simulink model "MX_Gateway"  (Simulink 9.0) (continued)
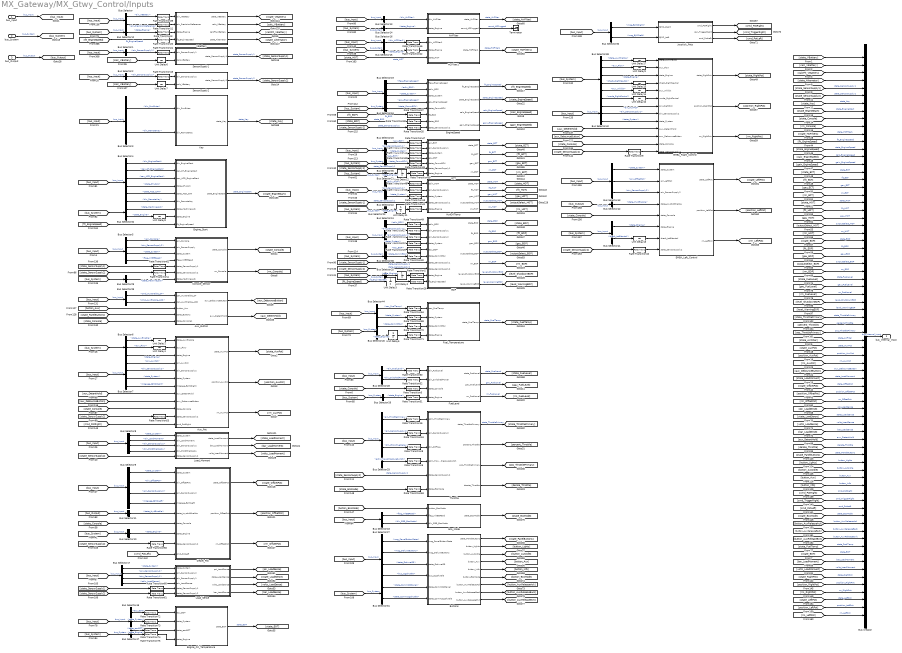
== system MX_Gateway/MX_Gtwy_Control/Inputs ==
  bus_Input : Inport
  bus_System : Inport  Port=2
  bus_Output : Inport  Port=3
  AirFilter : Subsystem  TreatAsAtomicUnit=on  SystemSampleTime=srate  RTWFileName=mod_Inputs
      settings: RTWSystemCode=Nonreusable function  RTWFileNameOpts=User specified
  Aux_Button : Subsystem
  Aux_Pot : Subsystem  TreatAsAtomicUnit=on  SystemSampleTime=srate  RTWFileName=mod_Inputs
      settings: RTWSystemCode=Nonreusable function  RTWFcnNameOpts=Use subsystem name  RTWFileNameOpts=User specified
  Bus Creator : BusCreator  Inputs=74
  Bus Selector : BusSelector  OutputSignals=bus_AnalogInputs.ain_VBattery,bus_AnalogInputs.ain_PrecisionReference
  Bus Selector1 : BusSelector  OutputSignals=bus_AnalogInputs.ain_SensorSupply1
  Bus Selector{10,12,14..15,21,23,25..26,47} (9 alike) : BusSelector  OutputSignals=state_Engine
  Bus Selector11 : BusSelector  OutputSignals=state_HydEnable
  Bus Selector13 : BusSelector  OutputSignals=bus_DigitalInputs.din_AirFilter
  Bus Selector16 : BusSelector  OutputSignals=bus_DigitalInputs.din_HCFilter1
  Bus Selector17 : BusSelector  OutputSignals=bus_Controller.state_System,bus_AnalogInputs.ain_SensorSupply2,bus_AnalogInputs.ain_SensorSupply1,bus_CombinedInputs.adin_LoadSense
  Bus Selector18 : BusSelector  OutputSignals=bus_Controller.flag_InitEERead,bus_CAN.bus_DeluxeG55.dlx_G55_EcoMode
  Bus Selector19 : BusSelector  OutputSignals=bus_FreqInputs.freq_EngineSpeed,bus_CombinedInputs.adin_EOP,bus_Controller.state_System,bus_CAN.bus_ECU.ecu_EngineSpeed
  Bus Selector2 : BusSelector  OutputSignals=bus_AnalogInputs.ain_SensorSupply2
  Bus Selector20 : BusSelector  OutputSignals=bus_AnalogInputs.ain_ECT,bus_AnalogInputs.ain_SensorSupply1,bus_Controller.state_System,bus_CAN.bus_ECU.ecu_ECT
  Bus Selector22 : BusSelector  OutputSignals=bus_CombinedInputs.adin_HOT,bus_AnalogInputs.ain_SensorSupply1,bus_Controller.state_System
  Bus Selector24 : BusSelector  OutputSignals=bus_CombinedInputs.adin_EOP,bus_AnalogInputs.ain_SensorSupply1,bus_AnalogInputs.ain_SensorSupply2,bus_Controller.state_System,bus_CAN.bus_ECU.ecu_EOP
  Bus Selector27 : BusSelector  OutputSignals=bus_CAN.bus_StdDisplay.msg_PanelButtonState,bus_CAN.bus_DeluxeG5.msg_DeluxeButtons,bus_CAN.bus_DeluxeG5.state_DeluxeG5,bus_CAN.bus_JogShuttle
  Bus Selector28 : BusSelector  OutputSignals=bus_AnalogInputs.ain_FuelLevel,bus_AnalogInputs.ain_SwitchedPower
  Bus Selector29 : BusSelector  OutputSignals=state_MachineLock
  Bus Selector3 : BusSelector  OutputSignals=bus_DigitalInputs.din_RunEnter,bus_DigitalInputs.din_RemoteKey
  Bus Selector30 : BusSelector  OutputSignals=bus_CAN.bus_CANJoystickRight.msg_BJM2Right,bus_CAN.bus_CANJoystickLeft.msg_BJM2Left
  Bus Selector31 : BusSelector  OutputSignals=state_CommStdPanel,state_CommJogShuttle
  Bus Selector32 : BusSelector  OutputSignals=state_MachineLock,state_Engine
  Bus Selector33 : BusSelector  OutputSignals=bus_AnalogInputs.ain_ThrottlePrimary,bus_AnalogInputs.ain_SensorSupply2,bus_DigitalInputs.din_GlowPlugRelay,bus_CombinedInputs.adin_TravelPressureSwitch
  Bus Selector34 : BusSelector  OutputSignals=state_CommECU
  Bus Selector35 : BusSelector  OutputSignals=state_CommECU
  Bus Selector36 : BusSelector  OutputSignals=state_CommECU
  Bus Selector38 : BusSelector  OutputSignals=state_AuxEnable,aux_Flow,state_Engine,check_RightNeutral,aux_Inhibit,mode_RightControl
  Bus Selector39 : BusSelector  OutputSignals=bus_CAN.bus_ServiceTool.cmd_AuxEnable_ST,bus_CAN.bus_ACD.cmd_AuxEnable_ACD
  Bus Selector4 : BusSelector  OutputSignals=bus_DigitalInputs.din_EngineStart,bus_CAN.bus_ServiceTool.can_ST_EngineStart,bus_CAN.bus_ACD.can_ACD_EngineStart,bus_CAN.bus_ServiceTool.state_ST_CAN,bus_CAN.bus_ACD.state_ACD_CAN,bus_DigitalInputs.din_RemoteKey
  Bus Selector40 : BusSelector  OutputSignals=bus_AnalogInputs.ain_AuxPot,bus_AnalogInputs.ain_SensorSupply2,bus_Controller.state_System
  Bus Selector41 : BusSelector  OutputSignals=state_Engine,check_LeftNeutral
  Bus Selector42 : BusSelector  OutputSignals=state_HydEnable
  Bus Selector43 : BusSelector  OutputSignals=bus_Controller.state_System,bus_AnalogInputs.ain_OffsetPot,bus_AnalogInputs.ain_SensorSupply2
  Bus Selector44 : BusSelector  OutputSignals=bus_CAN.bus_ECU.can_FuelTemp,bus_Controller.state_System
  Bus Selector45 : BusSelector  OutputSignals=state_CommECU,state_Engine
  Bus Selector46 : BusSelector  OutputSignals=bus_CAN.bus_ECU.ecu_EOT,bus_Controller.state_System,bus_CAN.bus_ECU.state_ecuECT
  Bus Selector5 : BusSelector  OutputSignals=bus_AnalogInputs.ain_Console,bus_AnalogInputs.ain_SensorSupply2,bus_Controller.state_System,bus_Controller.flag_InitEERead
  Bus Selector6 : BusSelector  OutputSignals=state_AuxEnable,aux_Flow,state_Engine
  Bus Selector7 : BusSelector  OutputSignals=bus_AnalogInputs.ain_AuxPot,bus_AnalogInputs.ain_SensorSupply2,bus_Controller.state_System,bus_CAN.bus_CANJoystickRight.msgAge_BJM2Right
  Bus Selector8 : BusSelector  OutputSignals=bus_Controller.state_System,bus_AnalogInputs.ain_LoadMoment,bus_AnalogInputs.ain_SensorSupply2,bus_DigitalInputs.din_OWDEnableSw
  Bus Selector9 : BusSelector  OutputSignals=bus_Controller.state_System,bus_AnalogInputs.ain_OffsetPot,bus_AnalogInputs.ain_SensorSupply2,bus_CAN.bus_CANJoystickLeft.msgAge_BJM2Left
  Buttons : Subsystem  TreatAsAtomicUnit=on  SystemSampleTime=srate  RTWFileName=mod_Inputs
      settings: RTWSystemCode=Nonreusable function  RTWFileNameOpts=User specified
  Console_Sensor : Subsystem  TreatAsAtomicUnit=on  SystemSampleTime=srate  RTWFileName=mod_Inputs
      settings: RTWSystemCode=Nonreusable function  RTWFcnNameOpts=Use subsystem name  RTWFileNameOpts=User specified
  ECT : Subsystem  TreatAsAtomicUnit=on  SystemSampleTime=srate100  RTWFileName=mod_Inputs
      settings: RTWSystemCode=Nonreusable function  RTWFcnNameOpts=Use subsystem name  RTWFileNameOpts=User specified
  EMEA_Left_Control : Subsystem  SystemSampleTime=srate  RTWFileName=mod_Inputs
      settings: RTWSystemCode=Nonreusable function  RTWFcnNameOpts=Use subsystem name  RTWFileNameOpts=User specified
  EMEA_Right_Control : Subsystem
  EOP : Subsystem  TreatAsAtomicUnit=on  SystemSampleTime=srate100  RTWFileName=mod_Inputs
      settings: RTWSystemCode=Nonreusable function  RTWFcnNameOpts=Use subsystem name  RTWFileNameOpts=User specified
  Eco_Mode : Subsystem  TreatAsAtomicUnit=on  RTWFileName=mod_Inputs
      settings: RTWSystemCode=Nonreusable function  RTWFileNameOpts=User specified
  EngineSpeed : Subsystem  TreatAsAtomicUnit=on  SystemSampleTime=srate  RTWFileName=mod_Inputs
      settings: RTWSystemCode=Nonreusable function  RTWFcnNameOpts=Use subsystem name  RTWFileNameOpts=User specified
  Engine_Oil_Temperature : Subsystem  TreatAsAtomicUnit=on  SystemSampleTime=srate100  RTWFileName=mod_Inputs
      settings: RTWSystemCode=Nonreusable function  RTWFcnNameOpts=Use subsystem name  RTWFileNameOpts=User specified
  Engine_Start : Subsystem  TreatAsAtomicUnit=on  SystemSampleTime=srate  RTWFcnName=Engine_Start  RTWFileName=mod_Inputs
      settings: RTWSystemCode=Nonreusable function  RTWFcnNameOpts=User specified  RTWFileNameOpts=User specified
  FuelLevel : Subsystem  TreatAsAtomicUnit=on  SystemSampleTime=srate100  RTWFileName=mod_Inputs
      settings: RTWSystemCode=Nonreusable function  RTWFileNameOpts=User specified
  Fuel_Temperature : Subsystem  TreatAsAtomicUnit=on  SystemSampleTime=srate100  RTWFileName=mod_Inputs
      settings: RTWSystemCode=Nonreusable function  RTWFcnNameOpts=Use subsystem name  RTWFileNameOpts=User specified
  HCFilter1 : Subsystem  TreatAsAtomicUnit=on  SystemSampleTime=srate100  RTWFileName=mod_Inputs
      settings: RTWSystemCode=Nonreusable function  RTWFileNameOpts=User specified
  HydOilTemp : Subsystem  TreatAsAtomicUnit=on  SystemSampleTime=srate100  RTWFileName=mod_Inputs
      settings: RTWSystemCode=Nonreusable function  RTWFcnNameOpts=Use subsystem name  RTWFileNameOpts=User specified
  Joystick_Pots : Subsystem  TreatAsAtomicUnit=on  SystemSampleTime=srate  RTWFileName=mod_Inputs
      settings: RTWSystemCode=Nonreusable function  RTWFileNameOpts=User specified
  Key : Subsystem  TreatAsAtomicUnit=on  SystemSampleTime=srate  RTWFileName=mod_Inputs
      settings: RTWSystemCode=Nonreusable function  RTWFcnNameOpts=Use subsystem name  RTWFileNameOpts=User specified
  Load_Moment : Subsystem  RTWFileName=mod_Inputs
      settings: RTWFcnNameOpts=Use subsystem name  RTWFileNameOpts=User specified
  Load_Sense : Subsystem  TreatAsAtomicUnit=on  SystemSampleTime=srate  RTWFileName=mod_Inputs
      settings: RTWSystemCode=Nonreusable function  RTWFcnNameOpts=Use subsystem name  RTWFileNameOpts=User specified
  Offset_Pot : Subsystem  TreatAsAtomicUnit=on  SystemSampleTime=srate  RTWFileName=mod_Inputs
      settings: RTWSystemCode=Nonreusable function  RTWFcnNameOpts=Use subsystem name  RTWFileNameOpts=User specified
  Rate Transition{2..6,8..17,19..20,22,24,27..35,37..39,41,46..53,56,59..62,64,69..75} (53 alike) : RateTransition  Integrity=off
  Rate Transition63 : RateTransition  Integrity=off  Deterministic=off
  SensorSupply1 : Subsystem  TreatAsAtomicUnit=on  SystemSampleTime=srate100  RTWFileName=mod_Inputs
      settings: RTWSystemCode=Nonreusable function  RTWFcnNameOpts=Use subsystem name  RTWFileNameOpts=User specified
  SensorSupply2 : Subsystem  TreatAsAtomicUnit=on  SystemSampleTime=srate100  RTWFileName=mod_Inputs
      settings: RTWSystemCode=Nonreusable function  RTWFcnNameOpts=Use subsystem name  RTWFileNameOpts=User specified
  Terminator : Terminator
  Throttle : Subsystem  TreatAsAtomicUnit=on  SystemSampleTime=srate100  RTWFileName=mod_Inputs
      settings: RTWSystemCode=Nonreusable function  RTWFileNameOpts=User specified
  Unit Delay{1..15,17} (16 alike) : UnitDelay  SampleTime=-1
      Unit Delay11 settings: AttributesFormatString=<tsample=%<SampleTime>>\n<initial=%<X0>>
      Unit Delay12 settings: AttributesFormatString=<tsample=%<SampleTime>>\n<initial=%<X0>>
      Unit Delay2 settings: AttributesFormatString=<tsample=%<SampleTime>>\n<initial=%<X0>>
      Unit Delay3 settings: AttributesFormatString=<tsample=%<SampleTime>>\n<initial=%<X0>>
      Unit Delay5 settings: AttributesFormatString=<tsample=%<SampleTime>>\n<initial=%<X0>>
      Unit Delay6 settings: AttributesFormatString=<tsample=%<SampleTime>>\n<initial=%<X0>>
      Unit Delay7 settings: AttributesFormatString=<tsample=%<SampleTime>>\n<initial=%<X0>>
  VBattery : Subsystem  TreatAsAtomicUnit=on  SystemSampleTime=srate100  RTWFileName=mod_Inputs
      settings: RTWSystemCode=Nonreusable function  RTWFcnNameOpts=Use subsystem name  RTWFileNameOpts=User specified
  bus_Internal_Input : Outport
  wires:
  SensorSupply2/1(state_SensorSupply2) -> [state_SensorSupply2]  "state_SensorSupply2"
  SensorSupply1/1(state_SensorSupply1) -> [state_SensorSupply1]  "state_SensorSupply1"
  Key/1(state_Key) -> [state_Key]  "state_Key"
  VBattery/1(state_VBattery) -> [state_VBattery]
  VBattery/2(calc_VBattery) -> [calc_VBattery]
  VBattery/3(calcFlt_VBattery) -> [calcFlt_VBattery]
  [flt_EngineSpeed] -> Rate Transition20/1  "flt_EngineSpeed"
  VBattery/4(state_Alternator) -> [state_Alternator]
  Engine_Start/1(state_EngineStart) -> [state_EngineStart]  "state_EngineStart"
  [bus_Input] -> Bus Selector/1  "bus_Input"
  Bus Selector/1 -> Rate Transition39/1  "<ain_VBattery>"
  [bus_Input] -> Bus Selector1/1  "bus_Input"
  Bus Selector1/1 -> Rate Transition24/1  "<ain_SensorSupply1>"
  [bus_Input] -> Bus Selector2/1  "bus_Input"
  Bus Selector2/1 -> Rate Transition15/1  "<ain_SensorSupply2>"
  [bus_Input] -> Bus Selector3/1  "bus_Input"
  Bus Selector3/1 -> Key/1(din_RunEnter)  "<din_RunEnter>"
  Bus Selector3/2 -> Key/2(din_RemoteKey)  "<din_RemoteKey>"
  [bus_Input] -> Bus Selector4/1  "bus_Input"
  Bus Selector4/1 -> Engine_Start/1(din_EngineStart)  "<din_EngineStart>"
  bus_Input/1 -> [bus_Input]  "bus_Input"
  Bus Creator/1 -> bus_Internal_Input/1  "bus_Internal_Input"
  [state_VBattery] -> Bus Creator/1
  [calc_VBattery] -> Bus Creator/2
  [calcFlt_VBattery] -> Bus Creator/3
  [state_Alternator] -> Bus Creator/4
  [state_SensorSupply1] -> Bus Creator/5  "state_SensorSupply1"
  [state_SensorSupply2] -> Bus Creator/6  "state_SensorSupply2"
  [state_Key] -> Bus Creator/7  "state_Key"
  [state_EngineStart] -> Bus Creator/8  "state_EngineStart"
  Console_Sensor/1(state_Console) -> [state_Console]
  Console_Sensor/2(rm_Console) -> [rm_Console]
  [bus_Input] -> Bus Selector5/1  "bus_Input"
  Bus Selector5/1 -> Console_Sensor/1(ain_Console)  "<ain_Console>"
  Bus Selector5/2 -> Console_Sensor/2(ain_SensorSupply)  "<ain_SensorSupply2>"
  HCFilter1/1(state_HCFilter1) -> [state_HCFilter1]  "state_HCFilter1"
  AirFilter/1(state_AirFilter) -> [state_AirFilter]  "state_AirFilter"
  Rate Transition22/1 -> HCFilter1/2(state_Engine)
  [state_HOT] -> HCFilter1/3(state_HOT)  "state_HOT"
  Bus Selector20/1 -> Rate Transition47/1  "<ain_ECT>"
  Bus Selector20/2 -> Rate Transition17/1  "<ain_SensorSupply1>"
  ECT/1(state_ECT) -> [state_ECT]  "state_ECT"
  ECT/3(gau_ECT) -> [gau_ECT]  "gau_ECT"
  Bus Selector20/3 -> Rate Transition14/1  "<state_System>"
  Bus Selector22/1 -> Rate Transition48/1  "<adin_HOT>"
  Bus Selector22/2 -> Rate Transition19/1  "<ain_SensorSupply1>"
  Bus Selector22/3 -> Rate Transition13/1  "<state_System>"
  EOP/3(gau_EOP) -> [gau_EOP]  "gau_EOP"
  ECT/2(flt_ECT) -> [flt_ECT]  "flt_ECT"
  HydOilTemp/1(state_HOT) -> [state_HOT]  "state_HOT"
  HydOilTemp/2(flt_HOT) -> [flt_HOT]  "flt_HOT"
  HydOilTemp/3(gau_HOT) -> [gau_HOT]  "gau_HOT"
  EOP/1(state_EOP) -> [state_EOP]  "state_EOP"
  EOP/2(flt_EOP) -> [flt_EOP]  "flt_EOP"
  EOP/4(outputSelect_EOP) -> [outputSelect_EOP]  "outputSelect_EOP"
  HydOilTemp/4(outputSelect_HOT) -> [outputSelect_HOT]  "outputSelect_HOT"
  EOP/5(rm_EOP) -> [rm_EOP]  "rm_EOP"
  [state_SensorSupply1] -> HydOilTemp/4(state_SensorSupply1)  "state_SensorSupply1"
  HydOilTemp/5(rm_HOT) -> [rm_HOT]  "rm_HOT"
  ECT/4(rm_ECT) -> [rm_ECT]  "rm_ECT"
  Unit Delay11/1 -> Rate Transition41/1
  Bus Selector23/1 -> Unit Delay11/1  "<state_Engine>"
  Rate Transition41/1 -> HydOilTemp/5(state_Engine)
  Unit Delay12/1 -> Rate Transition59/1
  Bus Selector21/1 -> Unit Delay12/1  "<state_Engine>"
  Rate Transition59/1 -> ECT/7(state_Engine)
  FuelLevel/1(state_FuelLevel) -> [state_FuelLevel]  "state_FuelLevel"
  FuelLevel/2(gau_FuelLevel) -> [gau_FuelLevel]  "gau_FuelLevel"
  FuelLevel/3(rm_FuelLevel) -> [rm_FuelLevel]  "rm_FuelLevel"
  [state_Console] -> Bus Creator/9
  [rm_Console] -> Bus Creator/10
  bus_System/1 -> [bus_System]  "bus_System"
  [state_HCFilter1] -> Bus Creator/11  "state_HCFilter1"
  [flt_EngineSpeed] -> Bus Creator/12  "flt_EngineSpeed"
  [state_EngineSpeed] -> Bus Creator/13  "state_EngineSpeed"
  [calc_EngineSpeed] -> Bus Creator/14  "calc_EngineSpeed"
  [gain_EngineSpeed] -> Bus Creator/15  "gain_EngineSpeed"
  [state_ECT] -> Bus Creator/16  "state_ECT"
  [flt_ECT] -> Bus Creator/17  "flt_ECT"
  [gau_ECT] -> Bus Creator/18  "gau_ECT"
  [rm_ECT] -> Bus Creator/19  "rm_ECT"
  [state_HOT] -> Bus Creator/20  "state_HOT"
  [flt_HOT] -> Bus Creator/21  "flt_HOT"
  [gau_HOT] -> Bus Creator/22  "gau_HOT"
  [outputSelect_HOT] -> Bus Creator/23  "outputSelect_HOT"
  [rm_HOT] -> Bus Creator/24  "rm_HOT"
  [state_EOP] -> Bus Creator/25  "state_EOP"
  [flt_EOP] -> Bus Creator/26  "flt_EOP"
  [gau_EOP] -> Bus Creator/27  "gau_EOP"
  [outputSelect_EOP] -> Bus Creator/28  "outputSelect_EOP"
  [rm_EOP] -> Bus Creator/29  "rm_EOP"
  [state_FuelLevel] -> Bus Creator/30  "state_FuelLevel"
  [gau_FuelLevel] -> Bus Creator/31  "gau_FuelLevel"
  [rm_FuelLevel] -> Bus Creator/32  "rm_FuelLevel"
  [bus_System] -> Bus Selector12/1  "bus_Input"
  Bus Selector12/1 -> Rate Transition3/1  "<state_Engine>"
  [bus_Input] -> Bus Selector13/1  "bus_Input"
  Bus Selector13/1 -> AirFilter/1(din_AirFilter)  "<din_AirFilter>"
  [bus_System] -> Bus Selector14/1  "bus_System"
  Bus Selector14/1 -> AirFilter/2(state_Engine)  "<state_Engine>"
  [bus_System] -> Bus Selector15/1  "bus_System"
  Bus Selector15/1 -> Rate Transition22/1  "<state_Engine>"
  [bus_Input] -> Bus Selector16/1  "bus_Input"
  Bus Selector16/1 -> Rate Transition46/1  "<din_HCFilter1>"
  [bus_Input] -> Bus Selector19/1  "bus_Input"
  [bus_Input] -> Bus Selector20/1  "bus_Input"
  [bus_System] -> Bus Selector21/1  "bus_System"
  [bus_Input] -> Bus Selector22/1  "bus_Input"
  [bus_System] -> Bus Selector23/1  "bus_System"
  [bus_Input] -> Bus Selector24/1  "bus_Input"
  Unit Delay2/1 -> Rate Transition2/1
  Bus Selector25/1 -> Unit Delay2/1  "<state_Engine>"
  [bus_System] -> Bus Selector25/1  "bus_System"
  [flt_EngineSpeed] -> Unit Delay3/1  "flt_EngineSpeed"
  Unit Delay3/1 -> Rate Transition28/1
  [bus_Input] -> Bus Selector28/1  "bus_Input"
  Bus Selector28/1 -> Rate Transition50/1  "<ain_FuelLevel>"
  Bus Selector28/2 -> Rate Transition52/1  "<ain_SwitchedPower>"
  [state_SensorSupply1] -> ECT/6(state_SensorSupply1)  "state_SensorSupply1"
  [state_SensorSupply2] -> Rate Transition4/1
  Bus Selector24/2 -> Rate Transition27/1  "<ain_SensorSupply1>"
  Bus Selector24/3 -> Rate Transition33/1  "<ain_SensorSupply2>"
  Bus Selector24/4 -> Rate Transition12/1  "<state_System>"
  Bus Selector5/3 -> Console_Sensor/3(state_System)  "<state_System>"
  [bus_System] -> Bus Selector32/1
  Rate Transition13/1 -> HydOilTemp/3(state_System)
  Rate Transition14/1 -> ECT/3(state_System)
  Bus Selector24/1 -> Rate Transition49/1  "<adin_EOP>"
  Bus Selector/2 -> Rate Transition11/1  "<ain_PrecisionReference>"
  Bus Selector32/1 -> Engine_Start/7(state_MachineLock)  "<state_MachineLock>"
  EngineSpeed/1(flt_EngineSpeed) -> [flt_EngineSpeed]  "flt_EngineSpeed"
  EngineSpeed/2(state_EngineSpeed) -> [state_EngineSpeed]  "state_EngineSpeed"
  EngineSpeed/3(calc_EngineSpeed) -> [calc_EngineSpeed]  "calc_EngineSpeed"
  EngineSpeed/4(gain_EngineSpeed) -> [gain_EngineSpeed]  "gain_EngineSpeed"
  EOP/6(level_ShutdownEOP) -> [level_ShutdownEOP]  "level_ShutdownEOP"
  EOP/7(level_WarningEOP) -> [level_WarningEOP]  "level_WarningEOP"
  Rate Transition12/1 -> EOP/4(state_System)
  [state_SensorSupply1] -> EOP/7(state_SensorSupply1)  "state_SensorSupply1"
  [state_SensorSupply2] -> EOP/8(state_SensorSupply2)  "state_SensorSupply2"
  Rate Transition2/1 -> EOP/9(state_Engine)
  Bus Selector20/4 -> Rate Transition32/1  "<ecu_ECT>"
  Bus Selector24/5 -> Rate Transition29/1  "<ecu_EOP>"
  Bus Selector19/1 -> EngineSpeed/1(freq_EngineSpeed)  "<freq_EngineSpeed>"
  Bus Selector19/2 -> EngineSpeed/2(adin_EOP)  "<adin_EOP>"
  Bus Selector19/3 -> EngineSpeed/3(state_System)  "<state_System>"
  [flt_EOP] -> Rate Transition37/1  "flt_EOP"
  [state_EOP] -> Rate Transition38/1  "state_EOP"
  Throttle/1(state_ThrottlePrimary) -> [state_ThrottlePrimary]  "state_ThrottlePrimary"
  [bus_Input] -> Bus Selector33/1  "bus_Input"
  Bus Selector33/1 -> Rate Transition16/1  "<ain_ThrottlePrimary>"
  [bus_System] -> Bus Selector34/1  "bus_System"
  Bus Selector34/1 -> EngineSpeed/5(state_CommECU)  "<state_CommECU>"
  [bus_System] -> Bus Selector35/1  "bus_System"
  Bus Selector35/1 -> Rate Transition31/1  "<state_CommECU>"
  [bus_System] -> Bus Selector36/1  "bus_System"
  Bus Selector36/1 -> Rate Transition30/1  "<state_CommECU>"
  [state_SensorSupply2] -> Rate Transition35/1  "state_SensorSupply2"
  [level_ShutdownEOP] -> Bus Creator/33  "level_ShutdownEOP"
  [level_WarningEOP] -> Bus Creator/34  "level_WarningEOP"
  Rate Transition28/1 -> EOP/10(flt_EngineSpeed)
  Rate Transition29/1 -> EOP/5(ecu_EOP)
  Rate Transition30/1 -> EOP/6(state_CommECU)
  Rate Transition31/1 -> ECT/5(state_CommECU)
  Rate Transition32/1 -> ECT/4(ecu_ECT)
  Rate Transition37/1 -> EngineSpeed/6(flt_EOP)
  Rate Transition38/1 -> EngineSpeed/7(state_EOP)
  Rate Transition4/1 -> Console_Sensor/6(state_SensorSupply2)
  Rate Transition20/1 -> VBattery/4(flt_EngineSpeed)
  Rate Transition3/1 -> VBattery/3(state_Engine)
  [state_ThrottlePrimary] -> Bus Creator/35  "state_ThrottlePrimary"
  [percent_Throttle] -> Bus Creator/36  "percent_Throttle"
  [pos_ThrottlePrimary] -> Bus Creator/37  "pos_ThrottlePrimary"
  [state_Console] -> Rate Transition5/1
  Rate Transition5/1 -> FuelLevel/3(state_Console)
  Load_Moment/1(state_LoadMoment) -> [state_LoadMoment]
  [bus_System] -> Bus Selector6/1
  [bus_Input] -> Bus Selector7/1  "bus_Input"
  Bus Selector6/1 -> Unit Delay1/1  "<state_AuxEnable>"
  Bus Selector7/1 -> Aux_Pot/4(ain_AuxPot)  "<ain_AuxPot>"
  Bus Selector7/2 -> Aux_Pot/5(ain_SensorSupply2)  "<ain_SensorSupply2>"
  Bus Selector7/3 -> Aux_Pot/6(state_System)  "<state_System>"
  Aux_Pot/1(state_AuxPot) -> [state_AuxPot]
  Aux_Pot/2(position_AuxPot) -> [position_AuxPot]
  Aux_Pot/3(rm_AuxPot) -> [rm_AuxPot]
  [bus_Input] -> Bus Selector8/1  "bus_Input"
  Bus Selector8/1 -> Load_Moment/1(state_System)  "<state_System>"
  Bus Selector8/2 -> Load_Moment/2(ain_LoadMoment)  "<ain_LoadMoment>"
  [bus_Input] -> Bus Selector9/1  "bus_Input"
  Unit Delay1/1 -> Aux_Pot/1(state_AuxEnable)
  Bus Selector9/1 -> Offset_Pot/1(state_System)  "<state_System>"
  Bus Selector9/2 -> Offset_Pot/2(ain_OffsetPot)  "<ain_OffsetPot>"
  [state_Console] -> Offset_Pot/6(state_Console)
  [bus_System] -> Bus Selector10/1  "bus_Input"
  Offset_Pot/1(state_OffsetPot) -> [state_OffsetPot]
  Offset_Pot/2(position_OffsetPot) -> [position_OffsetPot]
  Offset_Pot/3(rm_OffsetPot) -> [rm_OffsetPot]
  bus_Output/1 -> [bus_Output]  "bus_Output"
  [bus_Output] -> Bus Selector11/1  "bus_Input"
  Bus Selector11/1 -> Offset_Pot/5(state_JoystickEnable)  "<state_HydEnable>"
  [bus_Input] -> Bus Selector17/1  "bus_Input"
  Bus Selector17/1 -> Load_Sense/1(state_System)  "<state_System>"
  Bus Selector17/2 -> Load_Sense/2(ain_SensorSupply2)  "<ain_SensorSupply2>"
  Bus Selector17/3 -> Load_Sense/3(ain_SensorSupply1)  "<ain_SensorSupply1>"
  Bus Selector17/4 -> Load_Sense/4(ain_LoadSense)  "<adin_LoadSense>"
  Load_Sense/1(psi_LoadSense) -> [psi_LoadSense]
  Load_Sense/2(state_LoadSense) -> [state_LoadSense]
  Load_Sense/3(volts_LoadSense) -> [volts_LoadSense]
  Load_Sense/4(bar_LoadSense) -> [bar_LoadSense]
  Bus Selector9/3 -> Offset_Pot/3(ain_SensorSupply2)  "<ain_SensorSupply2>"
  Throttle/2(percent_Throttle) -> [percent_Throttle]
  Throttle/3(pos_ThrottlePrimary) -> [pos_ThrottlePrimary]
  [state_SensorSupply2] -> Throttle/5(state_SensorSupply2)  "state_SensorSupply2"
  Rate Transition16/1 -> Throttle/1(adin_ThrottlePrimary)
  Bus Selector33/2 -> Rate Transition34/1  "<ain_SensorSupply2>"
  [bus_System] -> Bus Selector26/1  "bus_System"
  Bus Selector26/1 -> Rate Transition51/1  "<state_Engine>"
  Rate Transition51/1 -> FuelLevel/4(state_Engine)
  Rate Transition11/1 -> VBattery/2(ain_PrecisionReference)
  Rate Transition24/1 -> SensorSupply1/1(ain_SensorSupply1)
  Rate Transition15/1 -> SensorSupply2/1(ain_SensorSupply2)
  Rate Transition17/1 -> ECT/2(ain_SensorSupply1)
  Rate Transition19/1 -> HydOilTemp/2(ain_SensorSupply1)
  Rate Transition27/1 -> EOP/2(ain_SensorSupply1)
  Rate Transition33/1 -> EOP/3(ain_SensorSupply2)
  Rate Transition34/1 -> Throttle/2(ain_SensorSupply2)
  Rate Transition39/1 -> VBattery/1(ain_VBattery)
  Rate Transition46/1 -> HCFilter1/1(din_HCFilter1)
  Rate Transition35/1 -> EngineSpeed/8(state_SensorSupply2)
  Rate Transition47/1 -> ECT/1(ain_ECT)
  Rate Transition48/1 -> HydOilTemp/1(adin_HOT)
  Rate Transition49/1 -> EOP/1(adin_EOP)
  Rate Transition50/1 -> FuelLevel/1(ain_FuelLevel)
  Rate Transition52/1 -> FuelLevel/2(ain_SwitchedPower)
  Bus Selector6/3 -> Aux_Pot/3(state_Engine)  "<state_Engine>"
  [state_Console] -> Aux_Pot/10(state_Console)
  [state_AirFilter] -> Bus Creator/38  "state_AirFilter"
  [state_AuxPot] -> Bus Creator/39  "state_AuxPot"
  [position_AuxPot] -> Bus Creator/40  "position_AuxPot"
  [rm_AuxPot] -> Bus Creator/41  "rm_AuxPot"
  [aux_DebounceButton] -> Bus Creator/42  "aux_DebounceButton"
  [state_OffsetPot] -> Bus Creator/44  "state_OffsetPot"
  [position_OffsetPot] -> Bus Creator/45  "position_OffsetPot"
  [rm_OffsetPot] -> Bus Creator/46  "rm_OffsetPot"
  [psi_LoadSense] -> Bus Creator/47  "psi_LoadSense"
  [state_LoadSense] -> Bus Creator/48  "state_LoadSense"
  [volts_LoadSense] -> Bus Creator/49  "volts_LoadSense"
  [bar_LoadSense] -> Bus Creator/50  "bar_LoadSense"
  [state_LoadMoment] -> Bus Creator/43  "state_LoadMoment"
  [aux_DetentHold] -> Bus Creator/51  "aux_DetentHold"
  Throttle/4(derate_Throttle) -> [derate_Throttle]
  [derate_Throttle] -> Bus Creator/52  "derate_Throttle"
  Bus Selector6/2 -> Unit Delay4/1  "<aux_Flow>"
  Unit Delay4/1 -> Aux_Pot/2(aux_Flow)
  Bus Selector33/3 -> Rate Transition8/1  "<din_GlowPlugRelay>"
  Rate Transition8/1 -> Throttle/3(din_DPFKey)
  [state_SensorSupply2] -> Rate Transition53/1
  Rate Transition53/1 -> Aux_Pot/11(state_SensorSupply2)
  [state_SensorSupply2] -> Rate Transition56/1
  Rate Transition56/1 -> Offset_Pot/8(state_SensorSupply2)
  [state_SensorSupply1] -> Rate Transition60/1
  [state_SensorSupply2] -> Rate Transition61/1
  Rate Transition60/1 -> Load_Sense/5(state_SensorSupply1)
  Rate Transition61/1 -> Load_Sense/6(state_SensorSupply2)
  [bus_Input] -> Bus Selector27/1  "bus_Input"
  Bus Selector27/1 -> Buttons/1(msg_PanelButtonState)  "<msg_PanelButtonState>"
  Bus Selector27/2 -> Buttons/2(msg_DeluxeButtons)  "<msg_DeluxeButtons>"
  Bus Selector27/3 -> Buttons/3(state_DeluxeG5)  "<state_DeluxeG5>"
  Buttons/1(state_PanelButtons) -> [state_PanelButtons]
  Buttons/4(button_Aux) -> [button_Aux]
  Buttons/3(button_AutoIdle) -> [button_AutoIdle]
  Buttons/2(button_Lights) -> [button_Lights]
  [aux_DebounceButton] -> Aux_Pot/9(aux_DebounceButton)
  [state_PanelButtons] -> Bus Creator/53  "state_PanelButtons"
  [button_Lights] -> Bus Creator/54  "button_Lights"
  [button_AutoIdle] -> Bus Creator/55  "button_AutoIdle"
  [button_Aux] -> Bus Creator/56  "button_Aux"
  Buttons/5(button_Info) -> [button_Info]
  [button_Info] -> Bus Creator/57  "button_Info"
  [bus_System] -> Bus Selector29/1
  Bus Selector29/1 -> Console_Sensor/7(state_MachineLock)  "<state_MachineLock>"
  [state_SensorSupply1] -> Rate Transition62/1
  Rate Transition62/1 -> Console_Sensor/5(state_SensorSupply1)
  [bus_Input] -> Bus Selector30/1  "bus_Input"
  Bus Selector30/1 -> Joystick_Pots/1(BJM2_Right)  "<msg_BJM2Right>"
  Joystick_Pots/1(cmd_PotRight) -> [cmd_PotRight]
  Bus Selector30/2 -> Joystick_Pots/2(BJM2_Left)  "<msg_BJM2Left>"
  Joystick_Pots/2(can_TriggerRight) -> [cmd_TriggerRight]
  Joystick_Pots/3(cmd_PotLeft) -> [cmd_PotLeft]
  [cmd_PotRight] -> Bus Creator/58  "cmd_PotRight"
  [cmd_TriggerRight] -> Bus Creator/59  "cmd_TriggerRight"
  [cmd_PotLeft] -> Bus Creator/60  "cmd_PotLeft"
  Bus Selector9/4 -> Offset_Pot/4(msgAge_BJM2Left)  "<msgAge_BJM2Left>"
  [cmd_PotLeft] -> Offset_Pot/9(cmd_PotLeft)
  [cmd_PotRight] -> Aux_Pot/12(cmd_PotRight)
  Bus Selector7/4 -> Aux_Pot/7(msgAge_BJM2Right)  "<msgAge_BJM2Right>"
  Bus Selector4/2 -> Engine_Start/2(can_ST_EngineStart)  "<can_ST_EngineStart>"
  Bus Selector4/3 -> Engine_Start/3(can_ACD_EngineStart)  "<can_ACD_EngineStart>"
  Bus Selector4/4 -> Engine_Start/4(state_ST_CAN)  "<state_ST_CAN>"
  Bus Selector4/5 -> Engine_Start/5(state_ACD_CAN)  "<state_ACD_CAN>"
  [state_EcoMode] -> Bus Creator/61  "state_EcoMode"
  Rate Transition63/1 -> Throttle/6(state_EcoMode)
  Eco_Mode/1(state_EcoMode) -> [state_EcoMode]
  [button_EcoMode] -> Eco_Mode/1(button_EcoMode)
  [state_EcoMode] -> Rate Transition63/1
  [button_AuxReleaseAck] -> Bus Creator/62  "button_AuxReleaseAck"
  [button_AuxReleaseBack] -> Bus Creator/63  "button_AuxReleaseBack"
  [button_AuxReleaseExit] -> Bus Creator/64  "button_AuxReleaseExit"
  Bus Selector19/4 -> EngineSpeed/4(ecu_EngineSpeed)  "<ecu_EngineSpeed>"
  Bus Selector4/6 -> Engine_Start/6(din_RemoteKey)  "<din_RemoteKey>"
  Buttons/6(button_EcoMode) -> [button_EcoMode]
  Buttons/7(button_AuxReleaseAck) -> [button_AuxReleaseAck]
  Buttons/8(button_AuxReleaseBack) -> [button_AuxReleaseBack]
  Buttons/9(button_AuxReleaseExit) -> [button_AuxReleaseExit]
  [bus_Input] -> Bus Selector44/1  "bus_Input"
  Bus Selector44/1 -> Rate Transition64/1  "<can_FuelTemp>"
  Bus Selector44/2 -> Rate Transition69/1  "<state_System>"
  [bus_System] -> Bus Selector45/1  "bus_System"
  Bus Selector45/1 -> Rate Transition70/1  "<state_CommECU>"
  Bus Selector45/2 -> Unit Delay5/1  "<state_Engine>"
  Fuel_Temperature/1(state_FuelTemp) -> [state_FuelTemp]  "state_FuelTemp"
  Rate Transition64/1 -> Fuel_Temperature/1(can_FuelTemp)
  Rate Transition69/1 -> Fuel_Temperature/2(state_System)
  Rate Transition70/1 -> Fuel_Temperature/3(state_CommECU)
  Rate Transition71/1 -> Fuel_Temperature/4(state_Engine)
  [state_FuelTemp] -> Bus Creator/65  "state_FuelTemp"
  Unit Delay5/1 -> Rate Transition71/1
  [bus_Input] -> Bus Selector46/1  "bus_Input"
  Bus Selector46/1 -> Rate Transition72/1  "<ecu_EOT>"
  Bus Selector46/2 -> Rate Transition73/1  "<state_System>"
  Engine_Oil_Temperature/1(state_EOT) -> [state_EOT]  "state_EOT"
  Rate Transition72/1 -> Engine_Oil_Temperature/1(ecu_EOT)
  Rate Transition73/1 -> Engine_Oil_Temperature/2(state_System)
  Bus Selector46/3 -> Rate Transition74/1  "<state_ecuECT>"
  Rate Transition74/1 -> Engine_Oil_Temperature/3(state_ecuECT)
  [bus_System] -> Bus Selector47/1  "bus_System"
  Bus Selector47/1 -> Rate Transition75/1  "<state_Engine>"
  Rate Transition75/1 -> Engine_Oil_Temperature/4(state_Engine)
  [state_EOT] -> Bus Creator/66  "state_EOT"
  [state_SensorSupply2] -> Load_Moment/5(state_SensorSupply2)
  Bus Selector8/3 -> Load_Moment/3(ain_SensorSupply2)  "<ain_SensorSupply2>"
  Load_Moment/2(bar_LoadMoment) -> [bar_LoadMoment]
  [bar_LoadMoment] -> Bus Creator/67  "bar_LoadMoment"
  Load_Moment/3(volts_LoadMoment) -> [volts_LoadMoment]
  [volts_LoadMoment] -> Bus Creator/68  "volts_LoadMoment"
  Bus Selector8/4 -> Load_Moment/4(din_OWDEnableSw)  "<din_OWDEnableSw>"
  [bus_Input] -> Bus Selector18/1
  Bus Selector18/1 -> Eco_Mode/2(flag_InitEERead)  "<flag_InitEERead>"
  [calc_VBattery] -> Unit Delay6/1
  Unit Delay6/1 -> SensorSupply1/2(calc_VBattery)
  [calc_VBattery] -> Unit Delay7/1
  Unit Delay7/1 -> SensorSupply2/2(calc_VBattery)
  AirFilter/2(count_AFPlugged) -> Terminator/1  "count_AFPlugged"
  Bus Selector32/2 -> Unit Delay8/1  "<state_Engine>"
  Unit Delay8/1 -> Engine_Start/8(state_Engine)
  Bus Selector33/4 -> Rate Transition6/1  "<adin_TravelPressureSwitch>"
  Rate Transition6/1 -> Throttle/4(adin_TravelPressureSwitch)
  Bus Selector27/4 -> Buttons/4(bus_JogShuttle)  "<bus_JogShuttle>"
  [bus_System] -> Bus Selector31/1  "bus_System"
  Bus Selector31/2 -> Buttons/6(state_CommJogShuttle)  "<state_CommJogShuttle>"
  Bus Selector31/1 -> Buttons/5(state_CommStdPanel)  "<state_CommStdPanel>"
  Bus Selector10/1 -> Offset_Pot/7(state_Engine)  "<state_Engine>"
  [button_Aux] -> Aux_Button/3(button_Aux)
  [state_PanelButtons] -> Aux_Button/4(state_Buttons)
  [state_Console] -> Aux_Button/5(state_Console1)
  [bus_Input] -> Bus Selector39/1  "bus_Input"
  Bus Selector39/1 -> Aux_Button/1(can_AuxEnable_ST)  "<cmd_AuxEnable_ST>"
  Bus Selector39/2 -> Aux_Button/2(can_AuxEnable_ACD)  "<cmd_AuxEnable_ACD>"
  Aux_Button/1(aux_DebounceButton) -> [aux_DebounceButton]
  Aux_Button/2(aux_DetentHold) -> [aux_DetentHold]
  [aux_DetentHold] -> Aux_Pot/8(aux_DetentHold)
  [bus_System] -> Bus Selector38/1
  [bus_Input] -> Bus Selector40/1  "bus_Input"
  Bus Selector38/1 -> Unit Delay17/1  "<state_AuxEnable>"
  Bus Selector38/2 -> Unit Delay9/1  "<aux_Flow>"
  [state_SensorSupply2] -> Rate Transition9/1
  [state_RightPot] -> Bus Creator/69  "state_RightPot"
  [position_RightPot] -> Bus Creator/70  "position_RightPot"
  [rm_RightPot] -> Bus Creator/71  "rm_RightPot"
  Bus Selector40/1 -> EMEA_Right_Control/7(ain_RightPot)  "<ain_AuxPot>"
  Bus Selector40/2 -> EMEA_Right_Control/8(ain_SensorSupply2)  "<ain_SensorSupply2>"
  [aux_DetentHold] -> EMEA_Right_Control/10(aux_DetentHold)
  [aux_DebounceButton] -> EMEA_Right_Control/11(aux_DebounceButton)
  [state_Console] -> EMEA_Right_Control/12(state_Console)
  Rate Transition9/1 -> EMEA_Right_Control/13(state_SensorSupply2)
  Bus Selector40/3 -> EMEA_Right_Control/9(state_System)  "<state_System>"
  [bus_Input] -> Bus Selector43/1  "bus_Input"
  Bus Selector43/1 -> EMEA_Left_Control/1(state_System)  "<state_System>"
  Bus Selector43/2 -> EMEA_Left_Control/2(ain_LeftPot)  "<ain_OffsetPot>"
  [state_Console] -> EMEA_Left_Control/5(state_Console)
  [bus_System] -> Bus Selector41/1  "bus_Input"
  EMEA_Left_Control/1(state_LeftPot) -> [state_LeftPot]
  EMEA_Left_Control/2(position_LeftPot) -> [position_LeftPot]
  EMEA_Left_Control/3(rm_LeftPot) -> [rm_LeftPot]
  [bus_Output] -> Bus Selector42/1  "bus_Input"
  Bus Selector42/1 -> EMEA_Left_Control/4(state_JoystickEnable)  "<state_HydEnable>"
  Bus Selector43/3 -> EMEA_Left_Control/3(ain_SensorSupply2)  "<ain_SensorSupply2>"
  [state_SensorSupply2] -> Rate Transition10/1
  Rate Transition10/1 -> EMEA_Left_Control/8(state_SensorSupply2)
  Bus Selector41/1 -> EMEA_Left_Control/6(state_Engine)  "<state_Engine>"
  [state_LeftPot] -> Bus Creator/72  "state_LeftPot"
  [position_LeftPot] -> Bus Creator/73  "position_LeftPot"
  [rm_LeftPot] -> Bus Creator/74  "rm_LeftPot"
  Bus Selector41/2 -> Unit Delay13/1  "<check_LeftNeutral>"
  Unit Delay13/1 -> EMEA_Left_Control/7(check_LeftNeutral)
  Bus Selector38/4 -> Unit Delay15/1  "<check_RightNeutral>"
  Bus Selector38/5 -> Unit Delay14/1  "<aux_Inhibit>"
  Bus Selector38/6 -> Unit Delay10/1  "<mode_RightControl>"
  EMEA_Right_Control/2(position_RightPot) -> [position_RightPot]
  EMEA_Right_Control/3(rm_RightPot) -> [rm_RightPot]
  Unit Delay10/1 -> EMEA_Right_Control/6(mode_RightControl)
  Unit Delay14/1 -> EMEA_Right_Control/5(aux_Inhibit)
  Unit Delay15/1 -> EMEA_Right_Control/4(check_RightNeutral)
  Unit Delay17/1 -> EMEA_Right_Control/1(state_AuxEnable)
  EMEA_Right_Control/1(state_RightPot) -> [state_RightPot]
  Bus Selector38/3 -> EMEA_Right_Control/3(state_Engine)  "<state_Engine>"
  Unit Delay9/1 -> EMEA_Right_Control/2(aux_Flow)
  [flt_EngineSpeed] -> Engine_Start/9(flt_EngineSpeed)
  Bus Selector18/2 -> Eco_Mode/3(dlx_G55_EcoMode)  "<dlx_G55_EcoMode>"
  Bus Selector5/4 -> Console_Sensor/4(flag_InitEERead)  "<flag_InitEERead>"
